simulink library "Garbage"  (R2023a)
settings: Solver=ode45  StartTime=0.0  StopTime=10.0  RelTol=1e-3  EnableMultiTasking=on
undefined symbols (not defined in the file or in its folder): c, ending_column_index, ending_row_index, framebuffer_16x1, framebuffer_2x2, framebuffer_4x4, framebuffer_8x8, HEIGHT, hot, POS_X, POS_Y, starting_column_index, starting_row_index, WIDTH
libraries: Memory_Blocks_Lib x459, simulink x235, simulink_extras x132, dspmtrx3 x116, Bit_Multiplexers_Lib x67, Bit_Demultiplexers_Lib x49, Byte_Multiplexers_Lib x40, Miscellaneous x38, Computer_Library x17, Decoders_Lib x14, commutil2 x12, Arithmetic_Operations_Lib x8
(part 16 of 26)

== system Garbage/NOT_USED_Text_Memory_16x1_Characters ==
  Col(1B) : Inport
  Write(1b) : Inport  Port=2
  Data_In(8x1B) : Inport  Port=3
  CLK : Inport  Port=4
  8B_Video_Memory{,1..15} (16 alike) : Memory_Blocks_Lib/Memory_Blocks_Lib/8B_Video_Memory
  Demux1 : Demux  Outputs=8
  Integer to Bit Converter4 : simulink/Logic and Bit Operations/Integer to Bit Converter  nbits=8  signedInputValues=Unsigned  bitOrder=MSB first  outDtype=uint8
      settings: LibrarySourceBlock=|
      | commutil2/Integer to Bit
      | Converter
  Select_Column : Decoders_Lib/Decoders_Lib/Decoder_4_to_16
  Terminator{1..3,24} (4 alike) : Terminator
  Row1_Col01(8x1B) : Outport
  Row1_Col02(8x1B) : Outport  Port=2
  Row1_Col03(8x1B) : Outport  Port=3
  Row1_Col04(8x1B) : Outport  Port=4
  Row1_Col05(8x1B) : Outport  Port=5
  Row1_Col06(8x1B) : Outport  Port=6
  Row1_Col07(8x1B) : Outport  Port=7
  Row1_Col08(8x1B) : Outport  Port=8
  Row1_Col09(8x1B) : Outport  Port=9
  Row1_Col10(8x1B) : Outport  Port=10
  Row1_Col11(8x1B) : Outport  Port=11
  Row1_Col12(8x1B) : Outport  Port=12
  Row1_Col13(8x1B) : Outport  Port=13
  Row1_Col14(8x1B) : Outport  Port=14
  Row1_Col15(8x1B) : Outport  Port=15
  Row1_Col16(8x1B) : Outport  Port=16
  wires:
  Col(1B)/1 -> Integer to Bit Converter4/1
  Integer to Bit Converter4/1 -> Demux1/1
  Data_In(8x1B)/1 -> 8B_Video_Memory6/4, 8B_Video_Memory5/4, 8B_Video_Memory4/4, 8B_Video_Memory3/4, 8B_Video_Memory2/4, 8B_Video_Memory/4, 8B_Video_Memory1/4, 8B_Video_Memory7/4, 8B_Video_Memory8/4, 8B_Video_Memory9/4, 8B_Video_Memory10/4, 8B_Video_Memory11/4, 8B_Video_Memory12/4, 8B_Video_Memory13/4, 8B_Video_Memory14/4, 8B_Video_Memory15/4
  8B_Video_Memory/1 -> Row1_Col01(8x1B)/1
  8B_Video_Memory1/1 -> Row1_Col02(8x1B)/1
  8B_Video_Memory2/1 -> Row1_Col03(8x1B)/1
  8B_Video_Memory3/1 -> Row1_Col04(8x1B)/1
  CLK/1 -> 8B_Video_Memory6/5, 8B_Video_Memory5/5, 8B_Video_Memory4/5, 8B_Video_Memory3/5, 8B_Video_Memory2/5, 8B_Video_Memory/5, 8B_Video_Memory1/5, 8B_Video_Memory7/5, 8B_Video_Memory8/5, 8B_Video_Memory9/5, 8B_Video_Memory10/5, 8B_Video_Memory11/5, 8B_Video_Memory12/5, 8B_Video_Memory13/5, 8B_Video_Memory14/5, 8B_Video_Memory15/5
  8B_Video_Memory4/1 -> Row1_Col05(8x1B)/1
  8B_Video_Memory5/1 -> Row1_Col06(8x1B)/1
  8B_Video_Memory6/1 -> Row1_Col07(8x1B)/1
  8B_Video_Memory7/1 -> Row1_Col08(8x1B)/1
  8B_Video_Memory8/1 -> Row1_Col09(8x1B)/1
  8B_Video_Memory9/1 -> Row1_Col10(8x1B)/1
  8B_Video_Memory10/1 -> Row1_Col11(8x1B)/1
  8B_Video_Memory11/1 -> Row1_Col12(8x1B)/1
  8B_Video_Memory12/1 -> Row1_Col13(8x1B)/1
  8B_Video_Memory13/1 -> Row1_Col14(8x1B)/1
  8B_Video_Memory14/1 -> Row1_Col15(8x1B)/1
  8B_Video_Memory15/1 -> Row1_Col16(8x1B)/1
  Demux1/5 -> Select_Column/1
  Demux1/6 -> Select_Column/2
  Demux1/8 -> Select_Column/4
  Demux1/7 -> Select_Column/3
  Select_Column/16 -> 8B_Video_Memory/2, 8B_Video_Memory/1
  Select_Column/15 -> 8B_Video_Memory1/2, 8B_Video_Memory1/1
  Write(1b)/1 -> 8B_Video_Memory/3, 8B_Video_Memory1/3, 8B_Video_Memory2/3, 8B_Video_Memory3/3, 8B_Video_Memory4/3, 8B_Video_Memory5/3, 8B_Video_Memory6/3, 8B_Video_Memory7/3, 8B_Video_Memory8/3, 8B_Video_Memory9/3, 8B_Video_Memory10/3, 8B_Video_Memory11/3, 8B_Video_Memory12/3, 8B_Video_Memory13/3, 8B_Video_Memory14/3, 8B_Video_Memory15/3
  Select_Column/14 -> 8B_Video_Memory2/2, 8B_Video_Memory2/1
  Select_Column/13 -> 8B_Video_Memory3/1, 8B_Video_Memory3/2
  Select_Column/12 -> 8B_Video_Memory4/1, 8B_Video_Memory4/2
  Select_Column/11 -> 8B_Video_Memory5/1, 8B_Video_Memory5/2
  Select_Column/10 -> 8B_Video_Memory6/1, 8B_Video_Memory6/2
  Select_Column/9 -> 8B_Video_Memory7/1, 8B_Video_Memory7/2
  Select_Column/8 -> 8B_Video_Memory8/1, 8B_Video_Memory8/2
  Select_Column/7 -> 8B_Video_Memory9/1, 8B_Video_Memory9/2
  Select_Column/6 -> 8B_Video_Memory10/1, 8B_Video_Memory10/2
  Select_Column/5 -> 8B_Video_Memory11/1, 8B_Video_Memory11/2
  Select_Column/4 -> 8B_Video_Memory12/1, 8B_Video_Memory12/2
  Select_Column/3 -> 8B_Video_Memory13/1, 8B_Video_Memory13/2
  Select_Column/2 -> 8B_Video_Memory14/1, 8B_Video_Memory14/2
  Select_Column/1 -> 8B_Video_Memory15/1, 8B_Video_Memory15/2
  Demux1/4 -> Terminator24/1
  Demux1/3 -> Terminator1/1
  Demux1/2 -> Terminator2/1
  Demux1/1 -> Terminator3/1

== system Garbage/NOT_USED_Text_Memory_2x2_Characters ==
  Row(1B) : Inport
  Col(1B) : Inport  Port=2
  Write(1b) : Inport  Port=3
  Data_In(8x1B) : Inport  Port=4
  CLK : Inport  Port=5
  8B_Video_Memory{,1..3} (4 alike) : Memory_Blocks_Lib/Memory_Blocks_Lib/8B_Video_Memory
  Constant8 : Constant  Value=0  OutDataTypeStr=uint8
  Constant9 : Constant  Value=0  OutDataTypeStr=uint8
  Integer to Bit Converter3 : commutil2/Integer to Bit Converter  nbits=1  signedInputValues=Unsigned  bitOrder=MSB first  outDtype=uint8
  Integer to Bit Converter4 : commutil2/Integer to Bit Converter  nbits=1  signedInputValues=Unsigned  bitOrder=MSB first  outDtype=uint8
  Select_Column : Decoders_Lib/Decoders_Lib/Decoder_2_to_4
  Select_Row : Decoders_Lib/Decoders_Lib/Decoder_2_to_4
  Terminator{1..2,4..5} (4 alike) : Terminator
  Row1_Col1(8x1B) : Outport
  Row1_Col2(8x1B) : Outport  Port=2
  Row2_Col1(8x1B) : Outport  Port=3
  Row2_Col2(8x1B) : Outport  Port=4
  wires:
  Row(1B)/1 -> Integer to Bit Converter3/1
  Integer to Bit Converter3/1 -> Select_Row/2
  Constant8/1 -> Select_Row/1
  Constant9/1 -> Select_Column/1
  Col(1B)/1 -> Integer to Bit Converter4/1
  Integer to Bit Converter4/1 -> Select_Column/2
  Data_In(8x1B)/1 -> 8B_Video_Memory/4, 8B_Video_Memory1/4, 8B_Video_Memory2/4, 8B_Video_Memory3/4
  8B_Video_Memory/1 -> Row1_Col1(8x1B)/1
  8B_Video_Memory1/1 -> Row1_Col2(8x1B)/1
  8B_Video_Memory2/1 -> Row2_Col1(8x1B)/1
  8B_Video_Memory3/1 -> Row2_Col2(8x1B)/1
  CLK/1 -> 8B_Video_Memory/5, 8B_Video_Memory1/5, 8B_Video_Memory2/5, 8B_Video_Memory3/5
  Select_Row/1 -> Terminator4/1
  Select_Row/2 -> Terminator5/1
  Select_Column/1 -> Terminator1/1
  Select_Column/2 -> Terminator2/1
  Select_Row/4 -> 8B_Video_Memory1/2, 8B_Video_Memory/2
  Select_Row/3 -> 8B_Video_Memory3/2, 8B_Video_Memory2/2
  Select_Column/4 -> 8B_Video_Memory2/1, 8B_Video_Memory/1
  Select_Column/3 -> 8B_Video_Memory3/1, 8B_Video_Memory1/1
  Write(1b)/1 -> 8B_Video_Memory/3, 8B_Video_Memory1/3, 8B_Video_Memory2/3, 8B_Video_Memory3/3

== system Garbage/NOT_USED_Text_Memory_4x4_Characters ==
  Row(1B) : Inport
  Col(1B) : Inport  Port=2
  Data_In(8x1B) : Inport  Port=3
  CLK : Inport  Port=4
  8B_Video_Memory{,1..15} (16 alike) : Memory_Blocks_Lib/Memory_Blocks_Lib/8B_Video_Memory
  Demux : Demux  Outputs=2
  Demux1 : Demux  Outputs=2
  Integer to Bit Converter3 : simulink/Logic and Bit Operations/Integer to Bit Converter  nbits=2  signedInputValues=Unsigned  bitOrder=MSB first  outDtype=uint8
      settings: LibrarySourceBlock=|
      | commutil2/Integer to Bit
      | Converter
  Integer to Bit Converter4 : simulink/Logic and Bit Operations/Integer to Bit Converter  nbits=2  signedInputValues=Unsigned  bitOrder=MSB first  outDtype=uint8
      settings: LibrarySourceBlock=|
      | commutil2/Integer to Bit
      | Converter
  Select_Column : Decoders_Lib/Decoders_Lib/Decoder_2_to_4
  Select_Row : Decoders_Lib/Decoders_Lib/Decoder_2_to_4
  Row1_Col1(8x1B) : Outport
  Row1_Col2(8x1B) : Outport  Port=2
  Row1_Col3(8x1B) : Outport  Port=3
  Row1_Col4(8x1B) : Outport  Port=4
  Row2_Col1(8x1B) : Outport  Port=5
  Row2_Col2(8x1B) : Outport  Port=6
  Row2_Col3(8x1B) : Outport  Port=7
  Row2_Col4(8x1B) : Outport  Port=8
  Row3_Col1(8x1B) : Outport  Port=9
  Row3_Col2(8x1B) : Outport  Port=10
  Row3_Col3(8x1B) : Outport  Port=11
  Row3_Col4(8x1B) : Outport  Port=12
  Row4_Col1(8x1B) : Outport  Port=13
  Row4_Col2(8x1B) : Outport  Port=14
  Row4_Col3(8x1B) : Outport  Port=15
  Row4_Col4(8x1B) : Outport  Port=16
  wires:
  Row(1B)/1 -> Integer to Bit Converter3/1
  Integer to Bit Converter3/1 -> Demux/1
  Col(1B)/1 -> Integer to Bit Converter4/1
  Integer to Bit Converter4/1 -> Demux1/1
  Data_In(8x1B)/1 -> 8B_Video_Memory15/3, 8B_Video_Memory14/3, 8B_Video_Memory13/3, 8B_Video_Memory12/3, 8B_Video_Memory11/3, 8B_Video_Memory10/3, 8B_Video_Memory9/3, 8B_Video_Memory8/3, 8B_Video_Memory6/3, 8B_Video_Memory5/3, 8B_Video_Memory4/3, 8B_Video_Memory2/3, 8B_Video_Memory1/3, 8B_Video_Memory/3, 8B_Video_Memory3/3, 8B_Video_Memory7/3
  8B_Video_Memory/1 -> Row1_Col1(8x1B)/1
  8B_Video_Memory1/1 -> Row1_Col2(8x1B)/1
  8B_Video_Memory2/1 -> Row1_Col3(8x1B)/1
  8B_Video_Memory3/1 -> Row1_Col4(8x1B)/1
  CLK/1 -> 8B_Video_Memory15/4, 8B_Video_Memory14/4, 8B_Video_Memory13/4, 8B_Video_Memory12/4, 8B_Video_Memory11/4, 8B_Video_Memory10/4, 8B_Video_Memory9/4, 8B_Video_Memory8/4, 8B_Video_Memory7/4, 8B_Video_Memory6/4, 8B_Video_Memory5/4, 8B_Video_Memory4/4, 8B_Video_Memory3/4, 8B_Video_Memory2/4, 8B_Video_Memory1/4, 8B_Video_Memory/4
  Select_Row/1 -> 8B_Video_Memory12/2, 8B_Video_Memory13/2, 8B_Video_Memory14/2, 8B_Video_Memory15/2
  Select_Row/2 -> 8B_Video_Memory8/2, 8B_Video_Memory9/2, 8B_Video_Memory10/2, 8B_Video_Memory11/2
  Select_Column/1 -> 8B_Video_Memory15/1, 8B_Video_Memory11/1, 8B_Video_Memory7/1, 8B_Video_Memory3/1
  Select_Column/2 -> 8B_Video_Memory14/1, 8B_Video_Memory10/1, 8B_Video_Memory6/1, 8B_Video_Memory2/1
  Select_Row/4 -> 8B_Video_Memory/2, 8B_Video_Memory1/2, 8B_Video_Memory2/2, 8B_Video_Memory3/2
  Select_Row/3 -> 8B_Video_Memory4/2, 8B_Video_Memory5/2, 8B_Video_Memory6/2, 8B_Video_Memory7/2
  Select_Column/4 -> 8B_Video_Memory12/1, 8B_Video_Memory8/1, 8B_Video_Memory4/1, 8B_Video_Memory/1
  Select_Column/3 -> 8B_Video_Memory13/1, 8B_Video_Memory9/1, 8B_Video_Memory5/1, 8B_Video_Memory1/1
  8B_Video_Memory4/1 -> Row2_Col1(8x1B)/1
  8B_Video_Memory5/1 -> Row2_Col2(8x1B)/1
  8B_Video_Memory6/1 -> Row2_Col3(8x1B)/1
  8B_Video_Memory7/1 -> Row2_Col4(8x1B)/1
  8B_Video_Memory8/1 -> Row3_Col1(8x1B)/1
  8B_Video_Memory9/1 -> Row3_Col2(8x1B)/1
  8B_Video_Memory10/1 -> Row3_Col3(8x1B)/1
  8B_Video_Memory11/1 -> Row3_Col4(8x1B)/1
  8B_Video_Memory12/1 -> Row4_Col1(8x1B)/1
  8B_Video_Memory13/1 -> Row4_Col2(8x1B)/1
  8B_Video_Memory14/1 -> Row4_Col3(8x1B)/1
  8B_Video_Memory15/1 -> Row4_Col4(8x1B)/1
  Demux/1 -> Select_Row/1
  Demux/2 -> Select_Row/2
  Demux1/1 -> Select_Column/1
  Demux1/2 -> Select_Column/2

== system Garbage/NOT_USED_Text_Memory_8x8_Characters ==
  Row(1B) : Inport
  Col(1B) : Inport  Port=2
  Data_In(8x1B) : Inport  Port=3
  CLK : Inport  Port=4
  8B_Video_Memory{,1..63} (64 alike) : Memory_Blocks_Lib/Memory_Blocks_Lib/8B_Video_Memory
  Demux : Demux  Outputs=3
  Demux1 : Demux  Outputs=3
  Integer to Bit Converter3 : simulink/Logic and Bit Operations/Integer to Bit Converter  nbits=3  signedInputValues=Unsigned  bitOrder=MSB first  outDtype=uint8
      settings: LibrarySourceBlock=|
      | commutil2/Integer to Bit
      | Converter
  Integer to Bit Converter4 : simulink/Logic and Bit Operations/Integer to Bit Converter  nbits=3  signedInputValues=Unsigned  bitOrder=MSB first  outDtype=uint8
      settings: LibrarySourceBlock=|
      | commutil2/Integer to Bit
      | Converter
  Select_Column : Decoders_Lib/Decoders_Lib/Decoder_3_to_8
  Select_Row : Decoders_Lib/Decoders_Lib/Decoder_3_to_8
  Row1_Col1(8x1B) : Outport
  Row1_Col2(8x1B) : Outport  Port=2
  Row1_Col3(8x1B) : Outport  Port=3
  Row1_Col4(8x1B) : Outport  Port=4
  Row2_Col1(8x1B) : Outport  Port=5
  Row2_Col2(8x1B) : Outport  Port=6
  Row2_Col3(8x1B) : Outport  Port=7
  Row2_Col4(8x1B) : Outport  Port=8
  Row3_Col1(8x1B) : Outport  Port=9
  Row3_Col2(8x1B) : Outport  Port=10
  Row3_Col3(8x1B) : Outport  Port=11
  Row3_Col4(8x1B) : Outport  Port=12
  Row4_Col1(8x1B) : Outport  Port=13
  Row4_Col2(8x1B) : Outport  Port=14
  Row4_Col3(8x1B) : Outport  Port=15
  Row4_Col4(8x1B) : Outport  Port=16
  Row1_Col1(8x1B)1 : Outport  Port=17
  Row1_Col2(8x1B)1 : Outport  Port=18
  Row1_Col3(8x1B)1 : Outport  Port=19
  Row1_Col4(8x1B)1 : Outport  Port=20
  Row2_Col1(8x1B)1 : Outport  Port=21
  Row2_Col2(8x1B)1 : Outport  Port=22
  Row2_Col3(8x1B)1 : Outport  Port=23
  Row2_Col4(8x1B)1 : Outport  Port=24
  Row3_Col1(8x1B)1 : Outport  Port=25
  Row3_Col2(8x1B)1 : Outport  Port=26
  Row3_Col3(8x1B)1 : Outport  Port=27
  Row3_Col4(8x1B)1 : Outport  Port=28
  Row4_Col1(8x1B)1 : Outport  Port=29
  Row4_Col2(8x1B)1 : Outport  Port=30
  Row4_Col3(8x1B)1 : Outport  Port=31
  Row4_Col4(8x1B)1 : Outport  Port=32
  Row1_Col1(8x1B)2 : Outport  Port=33
  Row1_Col2(8x1B)2 : Outport  Port=34
  Row1_Col3(8x1B)2 : Outport  Port=35
  Row1_Col4(8x1B)2 : Outport  Port=36
  Row2_Col1(8x1B)2 : Outport  Port=37
  Row2_Col2(8x1B)2 : Outport  Port=38
  Row2_Col3(8x1B)2 : Outport  Port=39
  Row2_Col4(8x1B)2 : Outport  Port=40
  Row3_Col1(8x1B)2 : Outport  Port=41
  Row3_Col2(8x1B)2 : Outport  Port=42
  Row3_Col3(8x1B)2 : Outport  Port=43
  Row3_Col4(8x1B)2 : Outport  Port=44
  Row4_Col1(8x1B)2 : Outport  Port=45
  Row4_Col2(8x1B)2 : Outport  Port=46
  Row4_Col3(8x1B)2 : Outport  Port=47
  Row4_Col4(8x1B)2 : Outport  Port=48
  Row1_Col1(8x1B)3 : Outport  Port=49
  Row1_Col2(8x1B)3 : Outport  Port=50
  Row1_Col3(8x1B)3 : Outport  Port=51
  Row1_Col4(8x1B)3 : Outport  Port=52
  Row2_Col1(8x1B)3 : Outport  Port=53
  Row2_Col2(8x1B)3 : Outport  Port=54
  Row2_Col3(8x1B)3 : Outport  Port=55
  Row2_Col4(8x1B)3 : Outport  Port=56
  Row3_Col1(8x1B)3 : Outport  Port=57
  Row3_Col2(8x1B)3 : Outport  Port=58
  Row3_Col3(8x1B)3 : Outport  Port=59
  Row3_Col4(8x1B)3 : Outport  Port=60
  Row4_Col1(8x1B)3 : Outport  Port=61
  Row4_Col2(8x1B)3 : Outport  Port=62
  Row4_Col3(8x1B)3 : Outport  Port=63
  Row4_Col4(8x1B)3 : Outport  Port=64
  wires:
  Row(1B)/1 -> Integer to Bit Converter3/1
  Integer to Bit Converter3/1 -> Demux/1
  Col(1B)/1 -> Integer to Bit Converter4/1
  Integer to Bit Converter4/1 -> Demux1/1
  8B_Video_Memory/1 -> Row1_Col1(8x1B)/1
  8B_Video_Memory1/1 -> Row1_Col2(8x1B)/1
  8B_Video_Memory2/1 -> Row1_Col3(8x1B)/1
  8B_Video_Memory3/1 -> Row1_Col4(8x1B)/1
  Select_Row/5 -> 8B_Video_Memory30/2, 8B_Video_Memory31/2, 8B_Video_Memory18/2, 8B_Video_Memory19/2, 8B_Video_Memory20/2, 8B_Video_Memory21/2, 8B_Video_Memory22/2, 8B_Video_Memory23/2
  Select_Row/6 -> 8B_Video_Memory16/2, 8B_Video_Memory17/2, 8B_Video_Memory24/2, 8B_Video_Memory25/2, 8B_Video_Memory26/2, 8B_Video_Memory27/2, 8B_Video_Memory28/2, 8B_Video_Memory29/2
  Select_Column/5 -> 8B_Video_Memory3/1, 8B_Video_Memory51/1, 8B_Video_Memory57/1, 8B_Video_Memory35/1, 8B_Video_Memory41/1, 8B_Video_Memory19/1, 8B_Video_Memory25/1, 8B_Video_Memory11/1
  Select_Column/6 -> 8B_Video_Memory2/1, 8B_Video_Memory50/1, 8B_Video_Memory56/1, 8B_Video_Memory34/1, 8B_Video_Memory40/1, 8B_Video_Memory18/1, 8B_Video_Memory24/1, 8B_Video_Memory10/1
  Select_Row/8 -> 8B_Video_Memory/2, 8B_Video_Memory1/2, 8B_Video_Memory2/2, 8B_Video_Memory3/2, 8B_Video_Memory4/2, 8B_Video_Memory5/2, 8B_Video_Memory6/2, 8B_Video_Memory7/2
  Select_Row/7 -> 8B_Video_Memory8/2, 8B_Video_Memory9/2, 8B_Video_Memory10/2, 8B_Video_Memory11/2, 8B_Video_Memory12/2, 8B_Video_Memory13/2, 8B_Video_Memory14/2, 8B_Video_Memory15/2
  Select_Column/8 -> 8B_Video_Memory/1, 8B_Video_Memory62/1, 8B_Video_Memory48/1, 8B_Video_Memory46/1, 8B_Video_Memory32/1, 8B_Video_Memory30/1, 8B_Video_Memory16/1, 8B_Video_Memory8/1
  Select_Column/7 -> 8B_Video_Memory1/1, 8B_Video_Memory63/1, 8B_Video_Memory49/1, 8B_Video_Memory47/1, 8B_Video_Memory33/1, 8B_Video_Memory31/1, 8B_Video_Memory17/1, 8B_Video_Memory9/1
  8B_Video_Memory4/1 -> Row2_Col1(8x1B)/1
  8B_Video_Memory5/1 -> Row2_Col2(8x1B)/1
  8B_Video_Memory6/1 -> Row2_Col3(8x1B)/1
  8B_Video_Memory7/1 -> Row2_Col4(8x1B)/1
  8B_Video_Memory8/1 -> Row3_Col1(8x1B)/1
  8B_Video_Memory9/1 -> Row3_Col2(8x1B)/1
  8B_Video_Memory10/1 -> Row3_Col3(8x1B)/1
  8B_Video_Memory11/1 -> Row3_Col4(8x1B)/1
  8B_Video_Memory12/1 -> Row4_Col1(8x1B)/1
  8B_Video_Memory13/1 -> Row4_Col2(8x1B)/1
  8B_Video_Memory14/1 -> Row4_Col3(8x1B)/1
  8B_Video_Memory15/1 -> Row4_Col4(8x1B)/1
  Demux/1 -> Select_Row/1
  Demux/2 -> Select_Row/2
  Demux1/1 -> Select_Column/1
  Demux1/2 -> Select_Column/2
  8B_Video_Memory16/1 -> Row1_Col1(8x1B)1/1
  8B_Video_Memory17/1 -> Row1_Col2(8x1B)1/1
  8B_Video_Memory24/1 -> Row1_Col3(8x1B)1/1
  8B_Video_Memory25/1 -> Row1_Col4(8x1B)1/1
  8B_Video_Memory26/1 -> Row2_Col1(8x1B)1/1
  8B_Video_Memory27/1 -> Row2_Col2(8x1B)1/1
  8B_Video_Memory28/1 -> Row2_Col3(8x1B)1/1
  8B_Video_Memory29/1 -> Row2_Col4(8x1B)1/1
  8B_Video_Memory30/1 -> Row3_Col1(8x1B)1/1
  8B_Video_Memory31/1 -> Row3_Col2(8x1B)1/1
  8B_Video_Memory18/1 -> Row3_Col3(8x1B)1/1
  8B_Video_Memory19/1 -> Row3_Col4(8x1B)1/1
  8B_Video_Memory20/1 -> Row4_Col1(8x1B)1/1
  8B_Video_Memory21/1 -> Row4_Col2(8x1B)1/1
  8B_Video_Memory22/1 -> Row4_Col3(8x1B)1/1
  8B_Video_Memory23/1 -> Row4_Col4(8x1B)1/1
  8B_Video_Memory32/1 -> Row1_Col1(8x1B)2/1
  8B_Video_Memory33/1 -> Row1_Col2(8x1B)2/1
  8B_Video_Memory40/1 -> Row1_Col3(8x1B)2/1
  8B_Video_Memory41/1 -> Row1_Col4(8x1B)2/1
  8B_Video_Memory42/1 -> Row2_Col1(8x1B)2/1
  8B_Video_Memory43/1 -> Row2_Col2(8x1B)2/1
  8B_Video_Memory44/1 -> Row2_Col3(8x1B)2/1
  8B_Video_Memory45/1 -> Row2_Col4(8x1B)2/1
  8B_Video_Memory46/1 -> Row3_Col1(8x1B)2/1
  8B_Video_Memory47/1 -> Row3_Col2(8x1B)2/1
  8B_Video_Memory34/1 -> Row3_Col3(8x1B)2/1
  8B_Video_Memory35/1 -> Row3_Col4(8x1B)2/1
  8B_Video_Memory36/1 -> Row4_Col1(8x1B)2/1
  8B_Video_Memory37/1 -> Row4_Col2(8x1B)2/1
  8B_Video_Memory38/1 -> Row4_Col3(8x1B)2/1
  8B_Video_Memory39/1 -> Row4_Col4(8x1B)2/1
  8B_Video_Memory48/1 -> Row1_Col1(8x1B)3/1
  8B_Video_Memory49/1 -> Row1_Col2(8x1B)3/1
  8B_Video_Memory56/1 -> Row1_Col3(8x1B)3/1
  8B_Video_Memory57/1 -> Row1_Col4(8x1B)3/1
  8B_Video_Memory58/1 -> Row2_Col1(8x1B)3/1
  8B_Video_Memory59/1 -> Row2_Col2(8x1B)3/1
  8B_Video_Memory60/1 -> Row2_Col3(8x1B)3/1
  8B_Video_Memory61/1 -> Row2_Col4(8x1B)3/1
  8B_Video_Memory62/1 -> Row3_Col1(8x1B)3/1
  8B_Video_Memory63/1 -> Row3_Col2(8x1B)3/1
  8B_Video_Memory50/1 -> Row3_Col3(8x1B)3/1
  8B_Video_Memory51/1 -> Row3_Col4(8x1B)3/1
  8B_Video_Memory52/1 -> Row4_Col1(8x1B)3/1
  8B_Video_Memory53/1 -> Row4_Col2(8x1B)3/1
  8B_Video_Memory54/1 -> Row4_Col3(8x1B)3/1
  8B_Video_Memory55/1 -> Row4_Col4(8x1B)3/1
  Data_In(8x1B)/1 -> 8B_Video_Memory55/3, 8B_Video_Memory54/3, 8B_Video_Memory53/3, 8B_Video_Memory52/3, 8B_Video_Memory51/3, 8B_Video_Memory50/3, 8B_Video_Memory63/3, 8B_Video_Memory62/3, 8B_Video_Memory61/3, 8B_Video_Memory60/3, 8B_Video_Memory59/3, 8B_Video_Memory58/3, 8B_Video_Memory57/3, 8B_Video_Memory56/3, 8B_Video_Memory49/3, 8B_Video_Memory48/3, 8B_Video_Memory39/3, 8B_Video_Memory38/3, 8B_Video_Memory37/3, 8B_Video_Memory36/3, 8B_Video_Memory35/3, 8B_Video_Memory34/3, 8B_Video_Memory47/3, 8B_Video_Memory46/3, 8B_Video_Memory45/3, 8B_Video_Memory44/3, 8B_Video_Memory43/3, 8B_Video_Memory42/3, 8B_Video_Memory41/3, 8B_Video_Memory40/3, 8B_Video_Memory33/3, 8B_Video_Memory32/3, 8B_Video_Memory23/3, 8B_Video_Memory22/3, 8B_Video_Memory21/3, 8B_Video_Memory20/3, 8B_Video_Memory19/3, 8B_Video_Memory18/3, 8B_Video_Memory31/3, 8B_Video_Memory30/3, 8B_Video_Memory29/3, 8B_Video_Memory28/3, 8B_Video_Memory27/3, 8B_Video_Memory26/3, 8B_Video_Memory25/3, 8B_Video_Memory24/3, 8B_Video_Memory17/3, 8B_Video_Memory16/3, 8B_Video_Memory15/3, 8B_Video_Memory14/3, 8B_Video_Memory13/3, 8B_Video_Memory12/3, 8B_Video_Memory11/3, 8B_Video_Memory10/3, 8B_Video_Memory9/3, 8B_Video_Memory8/3, 8B_Video_Memory6/3, 8B_Video_Memory5/3, 8B_Video_Memory4/3, 8B_Video_Memory2/3, 8B_Video_Memory1/3, 8B_Video_Memory/3, 8B_Video_Memory3/3, 8B_Video_Memory7/3
  CLK/1 -> 8B_Video_Memory55/4, 8B_Video_Memory54/4, 8B_Video_Memory53/4, 8B_Video_Memory52/4, 8B_Video_Memory51/4, 8B_Video_Memory50/4, 8B_Video_Memory63/4, 8B_Video_Memory62/4, 8B_Video_Memory61/4, 8B_Video_Memory60/4, 8B_Video_Memory59/4, 8B_Video_Memory58/4, 8B_Video_Memory57/4, 8B_Video_Memory56/4, 8B_Video_Memory49/4, 8B_Video_Memory48/4, 8B_Video_Memory39/4, 8B_Video_Memory38/4, 8B_Video_Memory37/4, 8B_Video_Memory36/4, 8B_Video_Memory35/4, 8B_Video_Memory34/4, 8B_Video_Memory47/4, 8B_Video_Memory46/4, 8B_Video_Memory45/4, 8B_Video_Memory44/4, 8B_Video_Memory43/4, 8B_Video_Memory42/4, 8B_Video_Memory41/4, 8B_Video_Memory40/4, 8B_Video_Memory33/4, 8B_Video_Memory32/4, 8B_Video_Memory23/4, 8B_Video_Memory22/4, 8B_Video_Memory21/4, 8B_Video_Memory20/4, 8B_Video_Memory19/4, 8B_Video_Memory18/4, 8B_Video_Memory31/4, 8B_Video_Memory30/4, 8B_Video_Memory29/4, 8B_Video_Memory28/4, 8B_Video_Memory27/4, 8B_Video_Memory26/4, 8B_Video_Memory25/4, 8B_Video_Memory24/4, 8B_Video_Memory17/4, 8B_Video_Memory16/4, 8B_Video_Memory15/4, 8B_Video_Memory14/4, 8B_Video_Memory13/4, 8B_Video_Memory12/4, 8B_Video_Memory11/4, 8B_Video_Memory10/4, 8B_Video_Memory9/4, 8B_Video_Memory8/4, 8B_Video_Memory7/4, 8B_Video_Memory6/4, 8B_Video_Memory5/4, 8B_Video_Memory4/4, 8B_Video_Memory3/4, 8B_Video_Memory2/4, 8B_Video_Memory1/4, 8B_Video_Memory/4
  Select_Row/4 -> 8B_Video_Memory32/2, 8B_Video_Memory33/2, 8B_Video_Memory40/2, 8B_Video_Memory41/2, 8B_Video_Memory42/2, 8B_Video_Memory43/2, 8B_Video_Memory44/2, 8B_Video_Memory45/2
  Select_Row/3 -> 8B_Video_Memory46/2, 8B_Video_Memory47/2, 8B_Video_Memory34/2, 8B_Video_Memory35/2, 8B_Video_Memory36/2, 8B_Video_Memory37/2, 8B_Video_Memory38/2, 8B_Video_Memory39/2
  Select_Row/2 -> 8B_Video_Memory48/2, 8B_Video_Memory49/2, 8B_Video_Memory56/2, 8B_Video_Memory57/2, 8B_Video_Memory58/2, 8B_Video_Memory60/2, 8B_Video_Memory61/2, 8B_Video_Memory59/2
  Select_Row/1 -> 8B_Video_Memory62/2, 8B_Video_Memory63/2, 8B_Video_Memory50/2, 8B_Video_Memory51/2, 8B_Video_Memory52/2, 8B_Video_Memory53/2, 8B_Video_Memory54/2, 8B_Video_Memory55/2
  Select_Column/4 -> 8B_Video_Memory52/1, 8B_Video_Memory58/1, 8B_Video_Memory36/1, 8B_Video_Memory42/1, 8B_Video_Memory20/1, 8B_Video_Memory26/1, 8B_Video_Memory12/1, 8B_Video_Memory4/1
  Select_Column/3 -> 8B_Video_Memory53/1, 8B_Video_Memory59/1, 8B_Video_Memory37/1, 8B_Video_Memory43/1, 8B_Video_Memory21/1, 8B_Video_Memory27/1, 8B_Video_Memory13/1, 8B_Video_Memory5/1
  Select_Column/2 -> 8B_Video_Memory54/1, 8B_Video_Memory60/1, 8B_Video_Memory38/1, 8B_Video_Memory44/1, 8B_Video_Memory22/1, 8B_Video_Memory28/1, 8B_Video_Memory14/1, 8B_Video_Memory6/1
  Select_Column/1 -> 8B_Video_Memory55/1, 8B_Video_Memory61/1, 8B_Video_Memory39/1, 8B_Video_Memory45/1, 8B_Video_Memory23/1, 8B_Video_Memory29/1, 8B_Video_Memory15/1, 8B_Video_Memory7/1
  Demux/3 -> Select_Row/3
  Demux1/3 -> Select_Column/3

== system Garbage/Nibble_Mux_16 ==
  note: (Bit_3)
  note: (Bit_2)
  note: Bit_Multiplexer_16_to_1
  note: (Bit_1)
  note: Bit_Multiplexer_16_to_1
  note: (Bit_0)
  note: Bit_Multiplexer_16_to_1
  note: Bit_Multiplexer_16_to_1
  note: Nibble_Multiplexer_16_to_1
  Select_15(1b) : Inport
  Select_14(1b) : Inport  Port=2
  Select_13(1b) : Inport  Port=3
  Select_12(1b) : Inport  Port=4
  Select_11(1b) : Inport  Port=5
  Select_10(1b) : Inport  Port=6
  Select_09(1b) : Inport  Port=7
  Select_08(1b) : Inport  Port=8
  Select_07(1b) : Inport  Port=9
  Select_06(1b) : Inport  Port=10
  Select_05(1b) : Inport  Port=11
  Select_04(1b) : Inport  Port=12
  Select_03(1b) : Inport  Port=13
  Select_02(1b) : Inport  Port=14
  Select_01(1b) : Inport  Port=15
  Select_00(1b) : Inport  Port=16
  Data_15(4b) : Inport  Port=17
  Data_14(4b) : Inport  Port=18
  Data_13(4b) : Inport  Port=19
  Data_12(4b) : Inport  Port=20
  Data_11(4b) : Inport  Port=21
  Data_10(4b) : Inport  Port=22
  Data_09(4b) : Inport  Port=23
  Data_08(4b) : Inport  Port=24
  Data_07(4b) : Inport  Port=25
  Data_06(4b) : Inport  Port=26
  Data_05(4b) : Inport  Port=27
  Data_04(4b) : Inport  Port=28
  Data_03(4b) : Inport  Port=29
  Data_02(4b) : Inport  Port=30
  Data_01(4b) : Inport  Port=31
  Data_00(4b) : Inport  Port=32
  Bus Creator : BusCreator  Inputs=4
  Bus Selector{1..16} (16 alike) : BusSelector  OutputSignals=b3,b2,b1,b0
  Logical Operator{37..52,54..69,71..86,88..103} (64 alike) : Logic  Operator=NAND  IconShape=distinctive  AllPortsSameDT=off  OutDataTypeStr=boolean
  Logical Operator{53,70,87,104} (4 alike) : Logic  Operator=NAND  Inputs=16  IconShape=distinctive  OutDataTypeStr=boolean
  Data(4b) : Outport
  wires:
  Bus Selector13/1 -> Logical Operator52/2  "<b3>"
  Bus Selector1/1 -> Logical Operator37/2  "<b3>"
  Bus Selector2/1 -> Logical Operator38/2  "<b3>"
  Bus Selector3/1 -> Logical Operator39/2  "<b3>"
  Bus Selector4/1 -> Logical Operator40/2  "<b3>"
  Bus Selector5/1 -> Logical Operator41/2  "<b3>"
  Bus Selector6/1 -> Logical Operator42/2  "<b3>"
  Bus Selector7/1 -> Logical Operator43/2  "<b3>"
  Bus Selector13/2 -> Logical Operator69/2  "<b2>"
  Bus Selector1/2 -> Logical Operator54/2  "<b2>"
  Bus Selector2/2 -> Logical Operator55/2  "<b2>"
  Bus Selector3/2 -> Logical Operator56/2  "<b2>"
  Bus Selector4/2 -> Logical Operator57/2  "<b2>"
  Bus Selector5/2 -> Logical Operator58/2  "<b2>"
  Bus Selector6/2 -> Logical Operator59/2  "<b2>"
  Bus Selector7/2 -> Logical Operator60/2  "<b2>"
  Bus Selector13/3 -> Logical Operator86/2  "<b1>"
  Bus Selector1/3 -> Logical Operator71/2  "<b1>"
  Bus Selector2/3 -> Logical Operator72/2  "<b1>"
  Bus Selector3/3 -> Logical Operator73/2  "<b1>"
  Bus Selector4/3 -> Logical Operator74/2  "<b1>"
  Bus Selector5/3 -> Logical Operator75/2  "<b1>"
  Bus Selector6/3 -> Logical Operator76/2  "<b1>"
  Bus Selector7/3 -> Logical Operator77/2  "<b1>"
  Bus Selector13/4 -> Logical Operator103/2  "<b0>"
  Bus Selector1/4 -> Logical Operator88/2  "<b0>"
  Bus Selector2/4 -> Logical Operator89/2  "<b0>"
  Bus Selector3/4 -> Logical Operator90/2  "<b0>"
  Bus Selector4/4 -> Logical Operator91/2  "<b0>"
  Bus Selector5/4 -> Logical Operator92/2  "<b0>"
  Bus Selector6/4 -> Logical Operator93/2  "<b0>"
  Bus Selector7/4 -> Logical Operator94/2  "<b0>"
  Logical Operator49/1 -> Logical Operator53/14
  Logical Operator43/1 -> Logical Operator53/8
  Logical Operator51/1 -> Logical Operator53/16
  Logical Operator46/1 -> Logical Operator53/11
  Logical Operator50/1 -> Logical Operator53/15
  Logical Operator37/1 -> Logical Operator53/2
  Logical Operator47/1 -> Logical Operator53/12
  Logical Operator39/1 -> Logical Operator53/4
  Logical Operator38/1 -> Logical Operator53/3
  Logical Operator44/1 -> Logical Operator53/9
  Logical Operator45/1 -> Logical Operator53/10
  Logical Operator40/1 -> Logical Operator53/5
  Logical Operator52/1 -> Logical Operator53/1
  Logical Operator42/1 -> Logical Operator53/7
  Logical Operator41/1 -> Logical Operator53/6
  Logical Operator48/1 -> Logical Operator53/13
  Logical Operator66/1 -> Logical Operator70/14
  Logical Operator60/1 -> Logical Operator70/8
  Logical Operator68/1 -> Logical Operator70/16
  Logical Operator63/1 -> Logical Operator70/11
  Logical Operator67/1 -> Logical Operator70/15
  Logical Operator54/1 -> Logical Operator70/2
  Logical Operator64/1 -> Logical Operator70/12
  Logical Operator56/1 -> Logical Operator70/4
  Logical Operator55/1 -> Logical Operator70/3
  Logical Operator61/1 -> Logical Operator70/9
  Logical Operator62/1 -> Logical Operator70/10
  Logical Operator57/1 -> Logical Operator70/5
  Logical Operator69/1 -> Logical Operator70/1
  Logical Operator59/1 -> Logical Operator70/7
  Logical Operator58/1 -> Logical Operator70/6
  Logical Operator65/1 -> Logical Operator70/13
  Logical Operator83/1 -> Logical Operator87/14
  Logical Operator77/1 -> Logical Operator87/8
  Logical Operator85/1 -> Logical Operator87/16
  Logical Operator80/1 -> Logical Operator87/11
  Logical Operator84/1 -> Logical Operator87/15
  Logical Operator71/1 -> Logical Operator87/2
  Logical Operator81/1 -> Logical Operator87/12
  Logical Operator73/1 -> Logical Operator87/4
  Logical Operator72/1 -> Logical Operator87/3
  Logical Operator78/1 -> Logical Operator87/9
  Logical Operator79/1 -> Logical Operator87/10
  Logical Operator74/1 -> Logical Operator87/5
  Logical Operator86/1 -> Logical Operator87/1
  Logical Operator76/1 -> Logical Operator87/7
  Logical Operator75/1 -> Logical Operator87/6
  Logical Operator82/1 -> Logical Operator87/13
  Logical Operator100/1 -> Logical Operator104/14
  Logical Operator94/1 -> Logical Operator104/8
  Logical Operator102/1 -> Logical Operator104/16
  Logical Operator97/1 -> Logical Operator104/11
  Logical Operator101/1 -> Logical Operator104/15
  Logical Operator88/1 -> Logical Operator104/2
  Logical Operator98/1 -> Logical Operator104/12
  Logical Operator90/1 -> Logical Operator104/4
  Logical Operator89/1 -> Logical Operator104/3
  Logical Operator95/1 -> Logical Operator104/9
  Logical Operator96/1 -> Logical Operator104/10
  Logical Operator91/1 -> Logical Operator104/5
  Logical Operator103/1 -> Logical Operator104/1
  Logical Operator93/1 -> Logical Operator104/7
  Logical Operator92/1 -> Logical Operator104/6
  Logical Operator99/1 -> Logical Operator104/13
  Logical Operator53/1 -> Bus Creator/1
  Logical Operator104/1 -> Bus Creator/4
  Logical Operator87/1 -> Bus Creator/3
  Logical Operator70/1 -> Bus Creator/2
  Bus Creator/1 -> Data(4b)/1
  Select_15(1b)/1 -> Logical Operator52/1, Logical Operator86/1, Logical Operator103/1, Logical Operator69/1
  Select_14(1b)/1 -> Logical Operator37/1, Logical Operator71/1, Logical Operator88/1, Logical Operator54/1
  Select_13(1b)/1 -> Logical Operator38/1, Logical Operator72/1, Logical Operator89/1, Logical Operator55/1
  Select_12(1b)/1 -> Logical Operator39/1, Logical Operator73/1, Logical Operator90/1, Logical Operator56/1
  Select_11(1b)/1 -> Logical Operator40/1, Logical Operator74/1, Logical Operator91/1, Logical Operator57/1
  Select_10(1b)/1 -> Logical Operator41/1, Logical Operator75/1, Logical Operator92/1, Logical Operator58/1
  Select_09(1b)/1 -> Logical Operator42/1, Logical Operator76/1, Logical Operator93/1, Logical Operator59/1
  Select_08(1b)/1 -> Logical Operator43/1, Logical Operator77/1, Logical Operator94/1, Logical Operator60/1
  Select_07(1b)/1 -> Logical Operator44/1, Logical Operator78/1, Logical Operator95/1, Logical Operator61/1
  Select_04(1b)/1 -> Logical Operator47/1, Logical Operator81/1, Logical Operator98/1, Logical Operator64/1
  Select_03(1b)/1 -> Logical Operator48/1, Logical Operator82/1, Logical Operator99/1, Logical Operator65/1
  Select_01(1b)/1 -> Logical Operator50/1, Logical Operator84/1, Logical Operator101/1, Logical Operator67/1
  Select_00(1b)/1 -> Logical Operator51/1, Logical Operator85/1, Logical Operator102/1, Logical Operator68/1
  Select_06(1b)/1 -> Logical Operator45/1, Logical Operator79/1, Logical Operator96/1, Logical Operator62/1
  Select_05(1b)/1 -> Logical Operator46/1, Logical Operator80/1, Logical Operator97/1, Logical Operator63/1
  Select_02(1b)/1 -> Logical Operator49/1, Logical Operator83/1, Logical Operator100/1, Logical Operator66/1
  Bus Selector9/1 -> Logical Operator44/2  "<b3>"
  Bus Selector9/2 -> Logical Operator61/2  "<b2>"
  Bus Selector9/3 -> Logical Operator78/2  "<b1>"
  Bus Selector9/4 -> Logical Operator95/2  "<b0>"
  Bus Selector8/1 -> Logical Operator45/2  "<b3>"
  Bus Selector8/2 -> Logical Operator62/2  "<b2>"
  Bus Selector8/3 -> Logical Operator79/2  "<b1>"
  Bus Selector8/4 -> Logical Operator96/2  "<b0>"
  Bus Selector10/1 -> Logical Operator46/2  "<b3>"
  Bus Selector10/2 -> Logical Operator63/2  "<b2>"
  Bus Selector10/3 -> Logical Operator80/2  "<b1>"
  Bus Selector10/4 -> Logical Operator97/2  "<b0>"
  Bus Selector11/1 -> Logical Operator47/2  "<b3>"
  Bus Selector11/2 -> Logical Operator64/2  "<b2>"
  Bus Selector11/4 -> Logical Operator98/2  "<b0>"
  Bus Selector11/3 -> Logical Operator81/2  "<b1>"
  Bus Selector12/1 -> Logical Operator48/2  "<b3>"
  Bus Selector12/2 -> Logical Operator65/2  "<b2>"
  Bus Selector12/3 -> Logical Operator82/2  "<b1>"
  Bus Selector12/4 -> Logical Operator99/2  "<b0>"
  Bus Selector14/1 -> Logical Operator49/2  "<b3>"
  Bus Selector14/2 -> Logical Operator66/2  "<b2>"
  Bus Selector14/3 -> Logical Operator83/2  "<b1>"
  Bus Selector14/4 -> Logical Operator100/2  "<b0>"
  Bus Selector15/1 -> Logical Operator50/2  "<b3>"
  Bus Selector15/2 -> Logical Operator67/2  "<b2>"
  Bus Selector15/3 -> Logical Operator84/2  "<b1>"
  Bus Selector15/4 -> Logical Operator101/2  "<b0>"
  Bus Selector16/1 -> Logical Operator51/2  "<b3>"
  Bus Selector16/2 -> Logical Operator68/2  "<b2>"
  Bus Selector16/3 -> Logical Operator85/2  "<b1>"
  Bus Selector16/4 -> Logical Operator102/2  "<b0>"
  Data_15(4b)/1 -> Bus Selector13/1
  Data_14(4b)/1 -> Bus Selector1/1
  Data_13(4b)/1 -> Bus Selector2/1
  Data_12(4b)/1 -> Bus Selector3/1
  Data_11(4b)/1 -> Bus Selector4/1
  Data_10(4b)/1 -> Bus Selector5/1
  Data_09(4b)/1 -> Bus Selector6/1
  Data_08(4b)/1 -> Bus Selector7/1
  Data_07(4b)/1 -> Bus Selector9/1
  Data_06(4b)/1 -> Bus Selector8/1
  Data_05(4b)/1 -> Bus Selector10/1
  Data_04(4b)/1 -> Bus Selector11/1
  Data_03(4b)/1 -> Bus Selector12/1
  Data_02(4b)/1 -> Bus Selector14/1
  Data_01(4b)/1 -> Bus Selector15/1
  Data_00(4b)/1 -> Bus Selector16/1

== system Garbage/Nibble_Mux_2 ==
  note: Nibble_Multiplexer_2_to_1
  note: Bit_Multiplexer_2_to_1
  note: ( Bit_3 )
  note: Bit_Multiplexer_2_to_1
  note: ( Bit_2 )
  note: Bit_Multiplexer_2_to_1
  note: ( Bit_1 )
  note: Bit_Multiplexer_2_to_1
  note: ( Bit_0 )
  Addr(1b) : Inport
  Data_1(4b) : Inport  Port=2
  Data_0(4b) : Inport  Port=3
  Bus Creator : BusCreator  Inputs=4
  Bus Selector1 : BusSelector  OutputSignals=b3,b2,b1,b0
  Bus Selector2 : BusSelector  OutputSignals=b3,b2,b1,b0
  Logical Operator{1..12} (12 alike) : Logic  Operator=NAND  IconShape=distinctive  AllPortsSameDT=off  OutDataTypeStr=boolean
  Logical Operator16 : Logic  Operator=NOT  Inputs=4  IconShape=distinctive  AllPortsSameDT=off  OutDataTypeStr=boolean
  Data(4b) : Outport
  wires:
  Data_0(4b)/1 -> Bus Selector2/1
  Bus Selector2/4 -> Logical Operator12/2  "<b0>"
  Bus Selector1/4 -> Logical Operator11/2  "<b0>"
  Bus Selector1/3 -> Logical Operator8/2  "<b1>"
  Bus Selector2/3 -> Logical Operator9/2  "<b1>"
  Bus Selector1/2 -> Logical Operator5/2  "<b2>"
  Bus Selector2/2 -> Logical Operator6/2  "<b2>"
  Bus Selector2/1 -> Logical Operator3/2  "<b3>"
  Bus Selector1/1 -> Logical Operator2/2  "<b3>"
  Logical Operator16/1 -> Logical Operator3/1, Logical Operator6/1, Logical Operator12/1, Logical Operator9/1
  Addr(1b)/1 -> Logical Operator16/1, Logical Operator2/1, Logical Operator5/1, Logical Operator11/1, Logical Operator8/1
  Logical Operator3/1 -> Logical Operator1/2
  Logical Operator2/1 -> Logical Operator1/1
  Logical Operator6/1 -> Logical Operator4/2
  Logical Operator5/1 -> Logical Operator4/1
  Logical Operator9/1 -> Logical Operator7/2
  Logical Operator8/1 -> Logical Operator7/1
  Logical Operator12/1 -> Logical Operator10/2
  Logical Operator11/1 -> Logical Operator10/1
  Bus Creator/1 -> Data(4b)/1
  Data_1(4b)/1 -> Bus Selector1/1
  Logical Operator1/1 -> Bus Creator/1
  Logical Operator4/1 -> Bus Creator/2
  Logical Operator7/1 -> Bus Creator/3
  Logical Operator10/1 -> Bus Creator/4

== system Garbage/Nibble_Mux_4 ==
  note: Nibble_Multiplexer_4_to_1
  note: Bit_Multiplexer_4_to_1
  note: (Bit_3)
  note: (Bit_2)
  note: Bit_Multiplexer_4_to_1
  note: (Bit_1)
  note: Bit_Multiplexer_4_to_1
  note: (Bit_0)
  note: Bit_Multiplexer_4_to_1
  Addr_1(1b) : Inport
  Addr_0(1b) : Inport  Port=2
  Data_3(4b) : Inport  Port=3
  Data_2(4b) : Inport  Port=4
  Data_1(4b) : Inport  Port=5
  Data_0(4b) : Inport  Port=6
  Bus Creator3 : BusCreator  Inputs=4
  Bus Selector{1..3,7} (4 alike) : BusSelector  OutputSignals=b3,b2,b1,b0
  Logical Operator{13,18..22,25..30,32..34,36} (16 alike) : Logic  Operator=NAND  Inputs=3  IconShape=distinctive  AllPortsSameDT=off  OutDataTypeStr=boolean
  Logical Operator14 : Logic  Operator=NOT  Inputs=4  IconShape=distinctive  AllPortsSameDT=off  OutDataTypeStr=boolean
  Logical Operator15 : Logic  Operator=NOT  Inputs=4  IconShape=distinctive  AllPortsSameDT=off  OutDataTypeStr=boolean
  Logical Operator{17,23..24,31} (4 alike) : Logic  Operator=NAND  Inputs=4  IconShape=distinctive  OutDataTypeStr=boolean
  Data(4b) : Outport
  wires:
  Bus Creator3/1 -> Data(4b)/1
  Logical Operator31/1 -> Bus Creator3/4
  Logical Operator24/1 -> Bus Creator3/3
  Logical Operator17/1 -> Bus Creator3/2
  Logical Operator23/1 -> Bus Creator3/1
  Bus Selector7/4 -> Logical Operator28/3  "<b0>"
  Bus Selector2/4 -> Logical Operator32/3  "<b0>"
  Bus Selector1/4 -> Logical Operator33/3  "<b0>"
  Bus Selector3/4 -> Logical Operator34/3  "<b0>"
  Bus Selector7/3 -> Logical Operator21/3  "<b1>"
  Bus Selector2/3 -> Logical Operator25/3  "<b1>"
  Bus Selector1/3 -> Logical Operator26/3  "<b1>"
  Bus Selector3/3 -> Logical Operator27/3  "<b1>"
  Bus Selector7/2 -> Logical Operator13/3  "<b2>"
  Bus Selector2/2 -> Logical Operator18/3  "<b2>"
  Bus Selector1/2 -> Logical Operator19/3  "<b2>"
  Bus Selector3/2 -> Logical Operator20/3  "<b2>"
  Bus Selector7/1 -> Logical Operator22/3  "<b3>"
  Bus Selector2/1 -> Logical Operator29/3  "<b3>"
  Bus Selector1/1 -> Logical Operator30/3  "<b3>"
  Bus Selector3/1 -> Logical Operator36/3  "<b3>"
  Logical Operator15/1 -> Logical Operator29/1, Logical Operator36/1, Logical Operator18/1, Logical Operator20/1, Logical Operator25/1, Logical Operator27/1, Logical Operator34/1, Logical Operator32/1
  Addr_0(1b)/1 -> Logical Operator15/1, Logical Operator22/1, Logical Operator30/1, Logical Operator13/1, Logical Operator19/1, Logical Operator21/1, Logical Operator26/1, Logical Operator28/1, Logical Operator33/1
  Logical Operator14/1 -> Logical Operator30/2, Logical Operator36/2, Logical Operator19/2, Logical Operator20/2, Logical Operator26/2, Logical Operator27/2, Logical Operator34/2, Logical Operator33/2
  Addr_1(1b)/1 -> Logical Operator14/1, Logical Operator22/2, Logical Operator29/2, Logical Operator13/2, Logical Operator18/2, Logical Operator21/2, Logical Operator25/2, Logical Operator28/2, Logical Operator32/2
  Data_3(4b)/1 -> Bus Selector7/1
  Data_2(4b)/1 -> Bus Selector2/1
  Data_1(4b)/1 -> Bus Selector1/1
  Data_0(4b)/1 -> Bus Selector3/1
  Logical Operator22/1 -> Logical Operator23/1
  Logical Operator36/1 -> Logical Operator23/4
  Logical Operator30/1 -> Logical Operator23/3
  Logical Operator29/1 -> Logical Operator23/2
  Logical Operator20/1 -> Logical Operator17/4
  Logical Operator13/1 -> Logical Operator17/1
  Logical Operator19/1 -> Logical Operator17/3
  Logical Operator18/1 -> Logical Operator17/2
  Logical Operator27/1 -> Logical Operator24/4
  Logical Operator21/1 -> Logical Operator24/1
  Logical Operator26/1 -> Logical Operator24/3
  Logical Operator25/1 -> Logical Operator24/2
  Logical Operator34/1 -> Logical Operator31/4
  Logical Operator28/1 -> Logical Operator31/1
  Logical Operator33/1 -> Logical Operator31/3
  Logical Operator32/1 -> Logical Operator31/2

== system Garbage/Nibble_Mux_8 ==
  note: Bit_Multiplexer_8_to_1
  note: (Bit_3)
  note: Bit_Multiplexer_8_to_1
  note: (Bit_2)
  note: Bit_Multiplexer_8_to_1
  note: (Bit_1)
  note: Bit_Multiplexer_8_to_1
  note: (Bit_0)
  note: Nibble_Multiplexer_8_to_1
  Addr_2(1b) : Inport
  Addr_1(1b) : Inport  Port=2
  Addr_0(1b) : Inport  Port=3
  Data_7(4b) : Inport  Port=4
  Data_6(4b) : Inport  Port=5
  Data_5(4b) : Inport  Port=6
  Data_4(4b) : Inport  Port=7
  Data_3(4b) : Inport  Port=8
  Data_2(4b) : Inport  Port=9
  Data_1(4b) : Inport  Port=10
  Data_0(4b) : Inport  Port=11
  Bus Creator : BusCreator  Inputs=4
  Bus Selector{1..7,13} (8 alike) : BusSelector  OutputSignals=b3,b2,b1,b0
  Logical Operator{,2..8,10,12,16,35,37..40,42,44..50,52..59} (32 alike) : Logic  Operator=NAND  Inputs=4  IconShape=distinctive  AllPortsSameDT=off  OutDataTypeStr=boolean
  Logical Operator11 : Logic  Operator=NOT  Inputs=4  IconShape=distinctive  AllPortsSameDT=off  OutDataTypeStr=boolean
  Logical Operator{9,41,51,60} (4 alike) : Logic  Operator=NAND  Inputs=8  IconShape=distinctive  OutDataTypeStr=boolean
  Logical Operator62 : Logic  Operator=NOT  Inputs=4  IconShape=distinctive  AllPortsSameDT=off  OutDataTypeStr=boolean
  Logical Operator64 : Logic  Operator=NOT  Inputs=4  IconShape=distinctive  AllPortsSameDT=off  OutDataTypeStr=boolean
  Data(4b) : Outport
  wires:
  Bus Creator/1 -> Data(4b)/1
  Logical Operator60/1 -> Bus Creator/4
  Logical Operator51/1 -> Bus Creator/3
  Logical Operator41/1 -> Bus Creator/2
  Logical Operator9/1 -> Bus Creator/1
  Bus Selector13/1 -> Logical Operator/1  "<b3>"
  Bus Selector1/1 -> Logical Operator2/1  "<b3>"
  Bus Selector2/1 -> Logical Operator3/1  "<b3>"
  Bus Selector3/1 -> Logical Operator4/1  "<b3>"
  Bus Selector4/1 -> Logical Operator5/1  "<b3>"
  Bus Selector5/1 -> Logical Operator6/1  "<b3>"
  Bus Selector6/1 -> Logical Operator7/1  "<b3>"
  Bus Selector7/1 -> Logical Operator8/1  "<b3>"
  Bus Selector13/2 -> Logical Operator10/1  "<b2>"
  Bus Selector1/2 -> Logical Operator12/1  "<b2>"
  Bus Selector2/2 -> Logical Operator16/1  "<b2>"
  Bus Selector3/2 -> Logical Operator35/1  "<b2>"
  Bus Selector4/2 -> Logical Operator37/1  "<b2>"
  Bus Selector5/2 -> Logical Operator38/1  "<b2>"
  Bus Selector6/2 -> Logical Operator39/1  "<b2>"
  Bus Selector7/2 -> Logical Operator40/1  "<b2>"
  Bus Selector13/3 -> Logical Operator42/1  "<b1>"
  Bus Selector1/3 -> Logical Operator44/1  "<b1>"
  Bus Selector2/3 -> Logical Operator45/1  "<b1>"
  Bus Selector3/3 -> Logical Operator46/1  "<b1>"
  Bus Selector4/3 -> Logical Operator47/1  "<b1>"
  Bus Selector5/3 -> Logical Operator48/1  "<b1>"
  Bus Selector6/3 -> Logical Operator49/1  "<b1>"
  Bus Selector7/3 -> Logical Operator50/1  "<b1>"
  Bus Selector13/4 -> Logical Operator52/1  "<b0>"
  Bus Selector1/4 -> Logical Operator53/1  "<b0>"
  Bus Selector2/4 -> Logical Operator54/1  "<b0>"
  Bus Selector3/4 -> Logical Operator55/1  "<b0>"
  Bus Selector4/4 -> Logical Operator56/1  "<b0>"
  Bus Selector5/4 -> Logical Operator57/1  "<b0>"
  Bus Selector6/4 -> Logical Operator58/1  "<b0>"
  Bus Selector7/4 -> Logical Operator59/1  "<b0>"
  Logical Operator62/1 -> Logical Operator5/3, Logical Operator6/3, Logical Operator7/3, Logical Operator8/3, Logical Operator37/3, Logical Operator38/3, Logical Operator39/3, Logical Operator40/3, Logical Operator47/3, Logical Operator48/3, Logical Operator49/3, Logical Operator50/3, Logical Operator56/3, Logical Operator57/3, Logical Operator59/3, Logical Operator58/3
  Logical Operator11/1 -> Logical Operator3/4, Logical Operator4/4, Logical Operator7/4, Logical Operator8/4, Logical Operator16/4, Logical Operator35/4, Logical Operator39/4, Logical Operator40/4, Logical Operator45/4, Logical Operator46/4, Logical Operator49/4, Logical Operator50/4, Logical Operator54/4, Logical Operator55/4, Logical Operator58/4, Logical Operator59/4
  Logical Operator64/1 -> Logical Operator2/2, Logical Operator4/2, Logical Operator6/2, Logical Operator8/2, Logical Operator12/2, Logical Operator35/2, Logical Operator38/2, Logical Operator40/2, Logical Operator44/2, Logical Operator46/2, Logical Operator48/2, Logical Operator50/2, Logical Operator53/2, Logical Operator55/2, Logical Operator59/2, Logical Operator57/2
  Addr_0(1b)/1 -> Logical Operator/2, Logical Operator3/2, Logical Operator5/2, Logical Operator7/2, Logical Operator10/2, Logical Operator16/2, Logical Operator37/2, Logical Operator39/2, Logical Operator42/2, Logical Operator45/2, Logical Operator47/2, Logical Operator49/2, Logical Operator52/2, Logical Operator54/2, Logical Operator58/2, Logical Operator56/2, Logical Operator64/1
  Addr_1(1b)/1 -> Logical Operator/4, Logical Operator2/4, Logical Operator5/4, Logical Operator6/4, Logical Operator10/4, Logical Operator12/4, Logical Operator37/4, Logical Operator38/4, Logical Operator42/4, Logical Operator44/4, Logical Operator47/4, Logical Operator48/4, Logical Operator52/4, Logical Operator53/4, Logical Operator56/4, Logical Operator57/4, Logical Operator11/1
  Addr_2(1b)/1 -> Logical Operator/3, Logical Operator2/3, Logical Operator3/3, Logical Operator4/3, Logical Operator10/3, Logical Operator12/3, Logical Operator16/3, Logical Operator35/3, Logical Operator42/3, Logical Operator44/3, Logical Operator45/3, Logical Operator46/3, Logical Operator52/3, Logical Operator53/3, Logical Operator54/3, Logical Operator55/3, Logical Operator62/1
  Data_7(4b)/1 -> Bus Selector13/1
  Data_6(4b)/1 -> Bus Selector1/1
  Data_0(4b)/1 -> Bus Selector7/1
  Data_1(4b)/1 -> Bus Selector6/1
  Data_2(4b)/1 -> Bus Selector5/1
  Data_3(4b)/1 -> Bus Selector4/1
  Data_4(4b)/1 -> Bus Selector3/1
  Data_5(4b)/1 -> Bus Selector2/1
  Logical Operator8/1 -> Logical Operator9/8
  Logical Operator2/1 -> Logical Operator9/2
  Logical Operator3/1 -> Logical Operator9/3
  Logical Operator5/1 -> Logical Operator9/5
  Logical Operator/1 -> Logical Operator9/1
  Logical Operator6/1 -> Logical Operator9/6
  Logical Operator7/1 -> Logical Operator9/7
  Logical Operator4/1 -> Logical Operator9/4
  Logical Operator40/1 -> Logical Operator41/8
  Logical Operator12/1 -> Logical Operator41/2
  Logical Operator16/1 -> Logical Operator41/3
  Logical Operator37/1 -> Logical Operator41/5
  Logical Operator10/1 -> Logical Operator41/1
  Logical Operator38/1 -> Logical Operator41/6
  Logical Operator39/1 -> Logical Operator41/7
  Logical Operator35/1 -> Logical Operator41/4
  Logical Operator50/1 -> Logical Operator51/8
  Logical Operator44/1 -> Logical Operator51/2
  Logical Operator45/1 -> Logical Operator51/3
  Logical Operator47/1 -> Logical Operator51/5
  Logical Operator42/1 -> Logical Operator51/1
  Logical Operator48/1 -> Logical Operator51/6
  Logical Operator49/1 -> Logical Operator51/7
  Logical Operator46/1 -> Logical Operator51/4
  Logical Operator59/1 -> Logical Operator60/8
  Logical Operator53/1 -> Logical Operator60/2
  Logical Operator54/1 -> Logical Operator60/3
  Logical Operator56/1 -> Logical Operator60/5
  Logical Operator52/1 -> Logical Operator60/1
  Logical Operator57/1 -> Logical Operator60/6
  Logical Operator58/1 -> Logical Operator60/7
  Logical Operator55/1 -> Logical Operator60/4

== system Garbage/Screen_40x25__ ==
  note: TODO:
      | - Add vertical distance between characters.
      | - Add horizontal distance between characters.
      | - Add distance from the screen edges.
  Data_3(256x1B) : Inport
  Data_2(256x1B) : Inport  Port=2
  Data_1(256x1B) : Inport  Port=3
  Data_0(256x1B) : Inport  Port=4
  Byte_2 : Constant  Value=c.char_7x7(:, :, 1:256)  OutDataTypeStr=uint8
  MATLAB Function : MATLAB Function  TreatAsAtomicUnit=on
  Matrix Viewer1 : dspobslib/Matrix Viewer  ImageParams=off  CMapStr=[0 0.3 0; 0 1 0]  AxisColorbar=on  AxisParams=off  AxisOrigin=Upper left corner  AxisTickMode=Auto  XTickRange=[0 1]  YTickRange=[0 1]  XLabel=X-axis  YLabel=Y-axis  ZLabel=Z-axis  FigPos=[1280 555 320*2 246*2]  AxisZoom=on
      settings: YMin=0  YMax=1
  Vector Concatenate : Concatenate  NumInputs=4
  wires:
  MATLAB Function/1(Framebuffer) -> Matrix Viewer1/1
  Data_3(256x1B)/1 -> Vector Concatenate/4
  Data_2(256x1B)/1 -> Vector Concatenate/3
  Data_1(256x1B)/1 -> Vector Concatenate/2
  Vector Concatenate/1 -> MATLAB Function/2(VideoMemory)
  Byte_2/1 -> MATLAB Function/1(CharTable)
  Data_0(256x1B)/1 -> Vector Concatenate/1

== system Garbage/Selector_00 ==
  Addr(1B) : Inport
  Bus Selector : BusSelector  OutputSignals=signal1,signal2,signal3,signal4,signal5,signal6,signal7,signal8
  Logical Operator{1..7,26} (8 alike) : Logic  Operator=NOT  Inputs=1  IconShape=distinctive  AllPortsSameDT=off  OutDataTypeStr=boolean
  Logical Operator9 : Logic  Inputs=8  IconShape=distinctive  AllPortsSameDT=off  OutDataTypeStr=boolean
  Select_0(1b) : Outport
  wires:
  Addr(1B)/1 -> Bus Selector/1
  Logical Operator26/1 -> Logical Operator9/1
  Bus Selector/1 -> Logical Operator26/1  "<signal1>"
  Logical Operator9/1 -> Select_0(1b)/1
  Logical Operator1/1 -> Logical Operator9/2
  Bus Selector/2 -> Logical Operator1/1  "<signal2>"
  Logical Operator2/1 -> Logical Operator9/3
  Bus Selector/3 -> Logical Operator2/1  "<signal3>"
  Logical Operator3/1 -> Logical Operator9/4
  Bus Selector/4 -> Logical Operator3/1  "<signal4>"
  Logical Operator4/1 -> Logical Operator9/5
  Bus Selector/5 -> Logical Operator4/1  "<signal5>"
  Logical Operator5/1 -> Logical Operator9/6
  Bus Selector/6 -> Logical Operator5/1  "<signal6>"
  Logical Operator6/1 -> Logical Operator9/7
  Bus Selector/7 -> Logical Operator6/1  "<signal7>"
  Logical Operator7/1 -> Logical Operator9/8
  Bus Selector/8 -> Logical Operator7/1  "<signal8>"
